simulink model "CCMSysServ"  (R2022b) (continued)
== system CCMSysServ/CCMSysServ_Runnable_sys == (continued)
  SysServCarCfgSts_SysServDUBlowerPwmSignSts_write : Outport  Port=106
      settings: EnsureOutportIsVirtual=on
  SysServCarCfgSts_SysServDUBlowerPwmSts_write : Outport  Port=107
      settings: EnsureOutportIsVirtual=on
  SysServCarCfgSts_SysServDUDCDefrostSts_write : Outport  Port=108
      settings: EnsureOutportIsVirtual=on
  SysServCarCfgSts_SysServDUDCFLMdeSts_write : Outport  Port=109
      settings: EnsureOutportIsVirtual=on
  SysServCarCfgSts_SysServDUDCModeSts_write : Outport  Port=110
      settings: EnsureOutportIsVirtual=on
  SysServCarCfgSts_SysServDUDCRecSts_write : Outport  Port=111
      settings: EnsureOutportIsVirtual=on
  SysServCarCfgSts_SysServDUDCSModeSts_write : Outport  Port=112
      settings: EnsureOutportIsVirtual=on
  SysServCarCfgSts_SysServDUDCSTLSts_write : Outport  Port=113
      settings: EnsureOutportIsVirtual=on
  SysServCarCfgSts_SysServDUDCTLSts_write : Outport  Port=114
      settings: EnsureOutportIsVirtual=on
  SysServCarCfgSts_SysServDUDCTRSts_write : Outport  Port=115
      settings: EnsureOutportIsVirtual=on
  SysServCarCfgSts_SysServDUFLFootSts_write : Outport  Port=116
      settings: EnsureOutportIsVirtual=on
  SysServCarCfgSts_SysServDUFLVentSts_write : Outport  Port=117
      settings: EnsureOutportIsVirtual=on
  SysServCarCfgSts_SysServDUFRFootSts_write : Outport  Port=118
      settings: EnsureOutportIsVirtual=on
  SysServCarCfgSts_SysServDUFRVentSts_write : Outport  Port=119
      settings: EnsureOutportIsVirtual=on
  SysServCarCfgSts_SysServDUIncarMotorSts_write : Outport  Port=120
      settings: EnsureOutportIsVirtual=on
  SysServCarCfgSts_SysServDULin1HBMFSts_write : Outport  Port=121
      settings: EnsureOutportIsVirtual=on
  SysServCarCfgSts_SysServDULin4HFAFSts_write : Outport  Port=122
      settings: EnsureOutportIsVirtual=on
  SysServCarCfgSts_SysServDUSFLFootSts_write : Outport  Port=123
      settings: EnsureOutportIsVirtual=on
  SysServCarCfgSts_SysServDUSFLVentSts_write : Outport  Port=124
      settings: EnsureOutportIsVirtual=on
  SysServCarCfgSts_SysServDUSFRFootSts_write : Outport  Port=125
      settings: EnsureOutportIsVirtual=on
  SysServCarCfgSts_SysServDUSFRVentSts_write : Outport  Port=126
      settings: EnsureOutportIsVirtual=on
  SysServCarCfgSts_SysServDUSnsrForFCmptmtTSts_write : Outport  Port=127
      settings: EnsureOutportIsVirtual=on
  SysServCarCfgSts_SysServDUSnsrForRair_write : Outport  Port=128
      settings: EnsureOutportIsVirtual=on
  SysServCarCfgSts_SysServDUSnsrForRCmptmtTSts_write : Outport  Port=129
      settings: EnsureOutportIsVirtual=on
  SysServEcuSlpDelayFinish_EcuSlpDelayFinishFlg_write : Outport  Port=130
      settings: EnsureOutportIsVirtual=on
  dp_LifeCycle_LifeCycle_write : Outport  Port=131
      settings: EnsureOutportIsVirtual=on
  wires:
  ComMPNCState_PNCState_read/1 -> Terminator_1/1
  ComMPNCState_VFCState_read/1 -> Terminator_2/1
  EcuKeepActv_EcuKeepActv_read/1 -> Terminator_3/1
  CarTiGlb_CarTiGlb_read/1 -> Terminator_4/1
  CarTiGlb_CarTiGlb_IsUpdated_read/1 -> Terminator_5/1
  ActrFrntReqForRowFirstLeModDistbn_ActrFrntReqForRowFirstLeModDistbn_read/1 -> Terminator_6/1
  CarCfg_CarCfg1_read/1 -> Terminator_7/1
  CarCfg_CarCfg10_read/1 -> Terminator_8/1
  CarCfg_CarCfg100_read/1 -> Terminator_9/1
  CarCfg_CarCfg1002_read/1 -> Terminator_10/1
  CarCfg_CarCfg1004_read/1 -> Terminator_11/1
  CarCfg_CarCfg1007_read/1 -> Terminator_12/1
  CarCfg_CarCfg101_read/1 -> Terminator_13/1
  CarCfg_CarCfg1011_read/1 -> Terminator_14/1
  CarCfg_CarCfg1017_read/1 -> Terminator_15/1
  CarCfg_CarCfg1018_read/1 -> Terminator_16/1
  CarCfg_CarCfg102_read/1 -> Terminator_17/1
  CarCfg_CarCfg103_read/1 -> Terminator_18/1
  CarCfg_CarCfg1034_read/1 -> Terminator_19/1
  CarCfg_CarCfg1038_read/1 -> Terminator_20/1
  CarCfg_CarCfg1039_read/1 -> Terminator_21/1
  CarCfg_CarCfg104_read/1 -> Terminator_22/1
  CarCfg_CarCfg1042_read/1 -> Terminator_23/1
  CarCfg_CarCfg1043_read/1 -> Terminator_24/1
  CarCfg_CarCfg1044_read/1 -> Terminator_25/1
  CarCfg_CarCfg1045_read/1 -> Terminator_26/1
  CarCfg_CarCfg1047_read/1 -> Terminator_27/1
  CarCfg_CarCfg105_read/1 -> Terminator_28/1
  CarCfg_CarCfg1052_read/1 -> Terminator_29/1
  CarCfg_CarCfg1057_read/1 -> Terminator_30/1
  CarCfg_CarCfg106_read/1 -> Terminator_31/1
  CarCfg_CarCfg1061_read/1 -> Terminator_32/1
  CarCfg_CarCfg1063_read/1 -> Terminator_33/1
  CarCfg_CarCfg1065_read/1 -> Terminator_34/1
  CarCfg_CarCfg107_read/1 -> Terminator_35/1
  CarCfg_CarCfg1075_read/1 -> Terminator_36/1
  CarCfg_CarCfg1077_read/1 -> Terminator_37/1
  CarCfg_CarCfg108_read/1 -> Terminator_38/1
  CarCfg_CarCfg1080_read/1 -> Terminator_39/1
  CarCfg_CarCfg1083_read/1 -> Terminator_40/1
  CarCfg_CarCfg1087_read/1 -> Terminator_41/1
  CarCfg_CarCfg1088_read/1 -> Terminator_42/1
  CarCfg_CarCfg109_read/1 -> Terminator_43/1
  CarCfg_CarCfg1090_read/1 -> Terminator_44/1
  CarCfg_CarCfg1096_read/1 -> Terminator_45/1
  CarCfg_CarCfg110_read/1 -> Terminator_46/1
  CarCfg_CarCfg1101_read/1 -> Terminator_47/1
  CarCfg_CarCfg1102_read/1 -> Terminator_48/1
  CarCfg_CarCfg1103_read/1 -> Terminator_49/1
  CarCfg_CarCfg1104_read/1 -> Terminator_50/1
  CarCfg_CarCfg111_read/1 -> Terminator_51/1
  CarCfg_CarCfg1111_read/1 -> Terminator_52/1
  CarCfg_CarCfg1112_read/1 -> Terminator_53/1
  CarCfg_CarCfg112_read/1 -> Terminator_54/1
  CarCfg_CarCfg113_read/1 -> Terminator_55/1
  CarCfg_CarCfg1130_read/1 -> Terminator_56/1
  CarCfg_CarCfg1134_read/1 -> Terminator_57/1
  CarCfg_CarCfg1136_read/1 -> Terminator_58/1
  CarCfg_CarCfg114_read/1 -> Terminator_59/1
  CarCfg_CarCfg115_read/1 -> Terminator_60/1
  CarCfg_CarCfg1154_read/1 -> Terminator_61/1
  CarCfg_CarCfg116_read/1 -> Terminator_62/1
  CarCfg_CarCfg1166_read/1 -> Terminator_63/1
  CarCfg_CarCfg117_read/1 -> Terminator_64/1
  CarCfg_CarCfg1170_read/1 -> Terminator_65/1
  CarCfg_CarCfg1171_read/1 -> Terminator_66/1
  CarCfg_CarCfg1172_read/1 -> Terminator_67/1
  CarCfg_CarCfg1173_read/1 -> Terminator_68/1
  CarCfg_CarCfg1174_read/1 -> Terminator_69/1
  CarCfg_CarCfg1175_read/1 -> Terminator_70/1
  CarCfg_CarCfg1177_read/1 -> Terminator_71/1
  CarCfg_CarCfg1178_read/1 -> Terminator_72/1
  CarCfg_CarCfg118_read/1 -> Terminator_73/1
  CarCfg_CarCfg1184_read/1 -> Terminator_74/1
  CarCfg_CarCfg1185_read/1 -> Terminator_75/1
  CarCfg_CarCfg1189_read/1 -> Terminator_76/1
  CarCfg_CarCfg119_read/1 -> Terminator_77/1
  CarCfg_CarCfg12_read/1 -> Terminator_78/1
  CarCfg_CarCfg120_read/1 -> Terminator_79/1
  CarCfg_CarCfg121_read/1 -> Terminator_80/1
  CarCfg_CarCfg1214_read/1 -> Terminator_81/1
  CarCfg_CarCfg1216_read/1 -> Terminator_82/1
  CarCfg_CarCfg1217_read/1 -> Terminator_83/1
  CarCfg_CarCfg1218_read/1 -> Terminator_84/1
  CarCfg_CarCfg1219_read/1 -> Terminator_85/1
  CarCfg_CarCfg122_read/1 -> Terminator_86/1
  CarCfg_CarCfg123_read/1 -> Terminator_87/1
  CarCfg_CarCfg1230_read/1 -> Terminator_88/1
  CarCfg_CarCfg1231_read/1 -> Terminator_89/1
  CarCfg_CarCfg1232_read/1 -> Terminator_90/1
  CarCfg_CarCfg1233_read/1 -> Terminator_91/1
  CarCfg_CarCfg1234_read/1 -> Terminator_92/1
  CarCfg_CarCfg124_read/1 -> Terminator_93/1
  CarCfg_CarCfg1247_read/1 -> Terminator_94/1
  CarCfg_CarCfg125_read/1 -> Terminator_95/1
  CarCfg_CarCfg1250_read/1 -> Terminator_96/1
  CarCfg_CarCfg1251_read/1 -> Terminator_97/1
  CarCfg_CarCfg1258_read/1 -> Terminator_98/1
  CarCfg_CarCfg1259_read/1 -> Terminator_99/1
  CarCfg_CarCfg126_read/1 -> Terminator_100/1
  CarCfg_CarCfg1260_read/1 -> Terminator_101/1
  CarCfg_CarCfg1261_read/1 -> Terminator_102/1
  CarCfg_CarCfg1264_read/1 -> Terminator_103/1
  CarCfg_CarCfg1266_read/1 -> Terminator_104/1
  CarCfg_CarCfg1267_read/1 -> Terminator_105/1
  CarCfg_CarCfg127_read/1 -> Terminator_106/1
  CarCfg_CarCfg1273_read/1 -> Terminator_107/1
  CarCfg_CarCfg1275_read/1 -> Terminator_108/1
  CarCfg_CarCfg1277_read/1 -> Terminator_109/1
  CarCfg_CarCfg1278_read/1 -> Terminator_110/1
  CarCfg_CarCfg1279_read/1 -> Terminator_111/1
  CarCfg_CarCfg128_read/1 -> Terminator_112/1
  CarCfg_CarCfg1283_read/1 -> Terminator_113/1
  CarCfg_CarCfg1285_read/1 -> Terminator_114/1
  CarCfg_CarCfg1286_read/1 -> Terminator_115/1
  CarCfg_CarCfg1287_read/1 -> Terminator_116/1
  CarCfg_CarCfg129_read/1 -> Terminator_117/1
  CarCfg_CarCfg13_read/1 -> Terminator_118/1
  CarCfg_CarCfg130_read/1 -> Terminator_119/1
  CarCfg_CarCfg131_read/1 -> Terminator_120/1
  CarCfg_CarCfg132_read/1 -> Terminator_121/1
  CarCfg_CarCfg133_read/1 -> Terminator_122/1
  CarCfg_CarCfg134_read/1 -> Terminator_123/1
  CarCfg_CarCfg135_read/1 -> Terminator_124/1
  CarCfg_CarCfg136_read/1 -> Terminator_125/1
  CarCfg_CarCfg137_read/1 -> Terminator_126/1
  CarCfg_CarCfg138_read/1 -> Terminator_127/1
  CarCfg_CarCfg139_read/1 -> Terminator_128/1
  CarCfg_CarCfg14_read/1 -> Terminator_129/1
  CarCfg_CarCfg140_read/1 -> Terminator_130/1
  CarCfg_CarCfg141_read/1 -> Terminator_131/1
  CarCfg_CarCfg142_read/1 -> Terminator_132/1
  CarCfg_CarCfg143_read/1 -> Terminator_133/1
  CarCfg_CarCfg144_read/1 -> Terminator_134/1
  CarCfg_CarCfg145_read/1 -> Terminator_135/1
  CarCfg_CarCfg146_read/1 -> Terminator_136/1
  CarCfg_CarCfg148_read/1 -> Terminator_137/1
  CarCfg_CarCfg149_read/1 -> Terminator_138/1
  CarCfg_CarCfg150_read/1 -> Terminator_139/1
  CarCfg_CarCfg151_read/1 -> Terminator_140/1
  CarCfg_CarCfg152_read/1 -> Terminator_141/1
  CarCfg_CarCfg153_read/1 -> Terminator_142/1
  CarCfg_CarCfg154_read/1 -> Terminator_143/1
  CarCfg_CarCfg155_read/1 -> Terminator_144/1
  CarCfg_CarCfg156_read/1 -> Terminator_145/1
  CarCfg_CarCfg157_read/1 -> Terminator_146/1
  CarCfg_CarCfg158_read/1 -> Terminator_147/1
  CarCfg_CarCfg159_read/1 -> Terminator_148/1
  CarCfg_CarCfg16_read/1 -> Terminator_149/1
  CarCfg_CarCfg160_read/1 -> Terminator_150/1
  CarCfg_CarCfg161_read/1 -> Terminator_151/1
  CarCfg_CarCfg162_read/1 -> Terminator_152/1
  CarCfg_CarCfg163_read/1 -> Terminator_153/1
  CarCfg_CarCfg164_read/1 -> Terminator_154/1
  CarCfg_CarCfg165_read/1 -> Terminator_155/1
  CarCfg_CarCfg166_read/1 -> Terminator_156/1
  CarCfg_CarCfg167_read/1 -> Terminator_157/1
  CarCfg_CarCfg168_read/1 -> Terminator_158/1
  CarCfg_CarCfg169_read/1 -> Terminator_159/1
  CarCfg_CarCfg17_read/1 -> Terminator_160/1
  CarCfg_CarCfg170_read/1 -> Terminator_161/1
  CarCfg_CarCfg171_read/1 -> Terminator_162/1
  CarCfg_CarCfg172_read/1 -> Terminator_163/1
  CarCfg_CarCfg173_read/1 -> Terminator_164/1
  CarCfg_CarCfg174_read/1 -> Terminator_165/1
  CarCfg_CarCfg175_read/1 -> Terminator_166/1
  CarCfg_CarCfg176_read/1 -> Terminator_167/1
  CarCfg_CarCfg177_read/1 -> Terminator_168/1
  CarCfg_CarCfg178_read/1 -> Terminator_169/1
  CarCfg_CarCfg179_read/1 -> Terminator_170/1
  CarCfg_CarCfg18_read/1 -> Terminator_171/1
  CarCfg_CarCfg180_read/1 -> Terminator_172/1
  CarCfg_CarCfg181_read/1 -> Terminator_173/1
  CarCfg_CarCfg182_read/1 -> Terminator_174/1
  CarCfg_CarCfg183_read/1 -> Terminator_175/1
  CarCfg_CarCfg184_read/1 -> Terminator_176/1
  CarCfg_CarCfg185_read/1 -> Terminator_177/1
  CarCfg_CarCfg186_read/1 -> Terminator_178/1
  CarCfg_CarCfg187_read/1 -> Terminator_179/1
  CarCfg_CarCfg188_read/1 -> Terminator_180/1
  CarCfg_CarCfg189_read/1 -> Terminator_181/1
  CarCfg_CarCfg19_read/1 -> Terminator_182/1
  CarCfg_CarCfg190_read/1 -> Terminator_183/1
  CarCfg_CarCfg191_read/1 -> Terminator_184/1
  CarCfg_CarCfg192_read/1 -> Terminator_185/1
  CarCfg_CarCfg193_read/1 -> Terminator_186/1
  CarCfg_CarCfg194_read/1 -> Terminator_187/1
  CarCfg_CarCfg195_read/1 -> Terminator_188/1
  CarCfg_CarCfg196_read/1 -> Terminator_189/1
  CarCfg_CarCfg197_read/1 -> Terminator_190/1
  CarCfg_CarCfg198_read/1 -> Terminator_191/1
  CarCfg_CarCfg199_read/1 -> Terminator_192/1
  CarCfg_CarCfg2_read/1 -> Terminator_193/1
  CarCfg_CarCfg20_read/1 -> Terminator_194/1
  CarCfg_CarCfg200_read/1 -> Terminator_195/1
  CarCfg_CarCfg201_read/1 -> Terminator_196/1
  CarCfg_CarCfg202_read/1 -> Terminator_197/1
  CarCfg_CarCfg203_read/1 -> Terminator_198/1
  CarCfg_CarCfg204_read/1 -> Terminator_199/1
  CarCfg_CarCfg205_read/1 -> Terminator_200/1
  CarCfg_CarCfg206_read/1 -> Terminator_201/1
  CarCfg_CarCfg207_read/1 -> Terminator_202/1
  CarCfg_CarCfg208_read/1 -> Terminator_203/1
  CarCfg_CarCfg209_read/1 -> Terminator_204/1
  CarCfg_CarCfg210_read/1 -> Terminator_205/1
  CarCfg_CarCfg211_read/1 -> Terminator_206/1
  CarCfg_CarCfg212_read/1 -> Terminator_207/1
  CarCfg_CarCfg213_read/1 -> Terminator_208/1
  CarCfg_CarCfg214_read/1 -> Terminator_209/1
  CarCfg_CarCfg215_read/1 -> Terminator_210/1
  CarCfg_CarCfg216_read/1 -> Terminator_211/1
  CarCfg_CarCfg217_read/1 -> Terminator_212/1
  CarCfg_CarCfg218_read/1 -> Terminator_213/1
  CarCfg_CarCfg219_read/1 -> Terminator_214/1
  CarCfg_CarCfg22_read/1 -> Terminator_215/1
  CarCfg_CarCfg220_read/1 -> Terminator_216/1
  CarCfg_CarCfg221_read/1 -> Terminator_217/1
  CarCfg_CarCfg222_read/1 -> Terminator_218/1
  CarCfg_CarCfg223_read/1 -> Terminator_219/1
  CarCfg_CarCfg224_read/1 -> Terminator_220/1
  CarCfg_CarCfg225_read/1 -> Terminator_221/1
  CarCfg_CarCfg226_read/1 -> Terminator_222/1
  CarCfg_CarCfg227_read/1 -> Terminator_223/1
  CarCfg_CarCfg228_read/1 -> Terminator_224/1
  CarCfg_CarCfg229_read/1 -> Terminator_225/1
  CarCfg_CarCfg23_read/1 -> Terminator_226/1
  CarCfg_CarCfg230_read/1 -> Terminator_227/1
  CarCfg_CarCfg231_read/1 -> Terminator_228/1
  CarCfg_CarCfg232_read/1 -> Terminator_229/1
  CarCfg_CarCfg235_read/1 -> Terminator_230/1
  CarCfg_CarCfg236_read/1 -> Terminator_231/1
  CarCfg_CarCfg237_read/1 -> Terminator_232/1
  CarCfg_CarCfg238_read/1 -> Terminator_233/1
  CarCfg_CarCfg239_read/1 -> Terminator_234/1
  CarCfg_CarCfg24_read/1 -> Terminator_235/1
  CarCfg_CarCfg240_read/1 -> Terminator_236/1
  CarCfg_CarCfg241_read/1 -> Terminator_237/1
  CarCfg_CarCfg242_read/1 -> Terminator_238/1
  CarCfg_CarCfg243_read/1 -> Terminator_239/1
  CarCfg_CarCfg244_read/1 -> Terminator_240/1
  CarCfg_CarCfg245_read/1 -> Terminator_241/1
  CarCfg_CarCfg246_read/1 -> Terminator_242/1
  CarCfg_CarCfg247_read/1 -> Terminator_243/1
  CarCfg_CarCfg248_read/1 -> Terminator_244/1
  CarCfg_CarCfg249_read/1 -> Terminator_245/1
  CarCfg_CarCfg25_read/1 -> Terminator_246/1
  CarCfg_CarCfg250_read/1 -> Terminator_247/1
  CarCfg_CarCfg253_read/1 -> Terminator_248/1
  CarCfg_CarCfg254_read/1 -> Terminator_249/1
  CarCfg_CarCfg255_read/1 -> Terminator_250/1
  CarCfg_CarCfg256_read/1 -> Terminator_251/1
  CarCfg_CarCfg257_read/1 -> Terminator_252/1
  CarCfg_CarCfg258_read/1 -> Terminator_253/1
  CarCfg_CarCfg259_read/1 -> Terminator_254/1
  CarCfg_CarCfg262_read/1 -> Terminator_255/1
  CarCfg_CarCfg263_read/1 -> Terminator_256/1
  CarCfg_CarCfg267_read/1 -> Terminator_257/1
  CarCfg_CarCfg268_read/1 -> Terminator_258/1
  CarCfg_CarCfg270_read/1 -> Terminator_259/1
  CarCfg_CarCfg271_read/1 -> Terminator_260/1
  CarCfg_CarCfg273_read/1 -> Terminator_261/1
  CarCfg_CarCfg274_read/1 -> Terminator_262/1
  CarCfg_CarCfg275_read/1 -> Terminator_263/1
  CarCfg_CarCfg28_read/1 -> Terminator_264/1
  CarCfg_CarCfg289_read/1 -> Terminator_265/1
  CarCfg_CarCfg290_read/1 -> Terminator_266/1
  CarCfg_CarCfg3_read/1 -> Terminator_267/1
  CarCfg_CarCfg30_read/1 -> Terminator_268/1
  CarCfg_CarCfg301_read/1 -> Terminator_269/1
  CarCfg_CarCfg302_read/1 -> Terminator_270/1
  CarCfg_CarCfg303_read/1 -> Terminator_271/1
  CarCfg_CarCfg304_read/1 -> Terminator_272/1
  CarCfg_CarCfg305_read/1 -> Terminator_273/1
  CarCfg_CarCfg307_read/1 -> Terminator_274/1
  CarCfg_CarCfg308_read/1 -> Terminator_275/1
  CarCfg_CarCfg309_read/1 -> Terminator_276/1
  CarCfg_CarCfg310_read/1 -> Terminator_277/1
  CarCfg_CarCfg311_read/1 -> Terminator_278/1
  CarCfg_CarCfg312_read/1 -> Terminator_279/1
  CarCfg_CarCfg313_read/1 -> Terminator_280/1
  CarCfg_CarCfg314_read/1 -> Terminator_281/1
  CarCfg_CarCfg315_read/1 -> Terminator_282/1
  CarCfg_CarCfg316_read/1 -> Terminator_283/1
  CarCfg_CarCfg317_read/1 -> Terminator_284/1
  CarCfg_CarCfg318_read/1 -> Terminator_285/1
  CarCfg_CarCfg319_read/1 -> Terminator_286/1
  CarCfg_CarCfg32_read/1 -> Terminator_287/1
  CarCfg_CarCfg321_read/1 -> Terminator_288/1
  CarCfg_CarCfg322_read/1 -> Terminator_289/1
  CarCfg_CarCfg323_read/1 -> Terminator_290/1
  CarCfg_CarCfg324_read/1 -> Terminator_291/1
  CarCfg_CarCfg325_read/1 -> Terminator_292/1
  CarCfg_CarCfg33_read/1 -> Terminator_293/1
  CarCfg_CarCfg335_read/1 -> Terminator_294/1
  CarCfg_CarCfg336_read/1 -> Terminator_295/1
  CarCfg_CarCfg337_read/1 -> Terminator_296/1
  CarCfg_CarCfg338_read/1 -> Terminator_297/1
  CarCfg_CarCfg339_read/1 -> Terminator_298/1
  CarCfg_CarCfg34_read/1 -> Terminator_299/1
  CarCfg_CarCfg340_read/1 -> Terminator_300/1
  CarCfg_CarCfg341_read/1 -> Terminator_301/1
  CarCfg_CarCfg342_read/1 -> Terminator_302/1
  CarCfg_CarCfg343_read/1 -> Terminator_303/1
  CarCfg_CarCfg344_read/1 -> Terminator_304/1
  CarCfg_CarCfg345_read/1 -> Terminator_305/1
  CarCfg_CarCfg346_read/1 -> Terminator_306/1
  CarCfg_CarCfg347_read/1 -> Terminator_307/1
  CarCfg_CarCfg348_read/1 -> Terminator_308/1
  CarCfg_CarCfg349_read/1 -> Terminator_309/1
  CarCfg_CarCfg35_read/1 -> Terminator_310/1
  CarCfg_CarCfg350_read/1 -> Terminator_311/1
  CarCfg_CarCfg351_read/1 -> Terminator_312/1
  CarCfg_CarCfg352_read/1 -> Terminator_313/1
  CarCfg_CarCfg353_read/1 -> Terminator_314/1
  CarCfg_CarCfg354_read/1 -> Terminator_315/1
  CarCfg_CarCfg355_read/1 -> Terminator_316/1
  CarCfg_CarCfg356_read/1 -> Terminator_317/1
  CarCfg_CarCfg357_read/1 -> Terminator_318/1
  CarCfg_CarCfg358_read/1 -> Terminator_319/1
  CarCfg_CarCfg359_read/1 -> Terminator_320/1
  CarCfg_CarCfg36_read/1 -> Terminator_321/1
  CarCfg_CarCfg360_read/1 -> Terminator_322/1
  CarCfg_CarCfg361_read/1 -> Terminator_323/1
  CarCfg_CarCfg362_read/1 -> Terminator_324/1
  CarCfg_CarCfg363_read/1 -> Terminator_325/1
  CarCfg_CarCfg364_read/1 -> Terminator_326/1
  CarCfg_CarCfg365_read/1 -> Terminator_327/1
  CarCfg_CarCfg366_read/1 -> Terminator_328/1
  CarCfg_CarCfg367_read/1 -> Terminator_329/1
  CarCfg_CarCfg368_read/1 -> Terminator_330/1
  CarCfg_CarCfg369_read/1 -> Terminator_331/1
  CarCfg_CarCfg37_read/1 -> Terminator_332/1
  CarCfg_CarCfg371_read/1 -> Terminator_333/1
  CarCfg_CarCfg372_read/1 -> Terminator_334/1
  CarCfg_CarCfg373_read/1 -> Terminator_335/1
  CarCfg_CarCfg374_read/1 -> Terminator_336/1
  CarCfg_CarCfg376_read/1 -> Terminator_337/1
  CarCfg_CarCfg38_read/1 -> Terminator_338/1
  CarCfg_CarCfg39_read/1 -> Terminator_339/1
  CarCfg_CarCfg4_read/1 -> Terminator_340/1
  CarCfg_CarCfg401_read/1 -> Terminator_341/1
  CarCfg_CarCfg407_read/1 -> Terminator_342/1
  CarCfg_CarCfg408_read/1 -> Terminator_343/1
  CarCfg_CarCfg409_read/1 -> Terminator_344/1
  CarCfg_CarCfg41_read/1 -> Terminator_345/1
  CarCfg_CarCfg410_read/1 -> Terminator_346/1
  CarCfg_CarCfg413_read/1 -> Terminator_347/1
  CarCfg_CarCfg414_read/1 -> Terminator_348/1
  CarCfg_CarCfg415_read/1 -> Terminator_349/1
  CarCfg_CarCfg42_read/1 -> Terminator_350/1
  CarCfg_CarCfg43_read/1 -> Terminator_351/1
  CarCfg_CarCfg44_read/1 -> Terminator_352/1
  CarCfg_CarCfg441_read/1 -> Terminator_353/1
  CarCfg_CarCfg449_read/1 -> Terminator_354/1
  CarCfg_CarCfg45_read/1 -> Terminator_355/1
  CarCfg_CarCfg450_read/1 -> Terminator_356/1
  CarCfg_CarCfg451_read/1 -> Terminator_357/1
  CarCfg_CarCfg452_read/1 -> Terminator_358/1
  CarCfg_CarCfg453_read/1 -> Terminator_359/1
  CarCfg_CarCfg454_read/1 -> Terminator_360/1
  CarCfg_CarCfg455_read/1 -> Terminator_361/1
  CarCfg_CarCfg456_read/1 -> Terminator_362/1
  CarCfg_CarCfg457_read/1 -> Terminator_363/1
  CarCfg_CarCfg458_read/1 -> Terminator_364/1
  CarCfg_CarCfg459_read/1 -> Terminator_365/1
  CarCfg_CarCfg46_read/1 -> Terminator_366/1
  CarCfg_CarCfg460_read/1 -> Terminator_367/1
  CarCfg_CarCfg461_read/1 -> Terminator_368/1
  CarCfg_CarCfg462_read/1 -> Terminator_369/1
  CarCfg_CarCfg463_read/1 -> Terminator_370/1
  CarCfg_CarCfg464_read/1 -> Terminator_371/1
  CarCfg_CarCfg465_read/1 -> Terminator_372/1
  CarCfg_CarCfg466_read/1 -> Terminator_373/1
  CarCfg_CarCfg467_read/1 -> Terminator_374/1
  CarCfg_CarCfg468_read/1 -> Terminator_375/1
  CarCfg_CarCfg469_read/1 -> Terminator_376/1
  CarCfg_CarCfg47_read/1 -> Terminator_377/1
  CarCfg_CarCfg470_read/1 -> Terminator_378/1
  CarCfg_CarCfg471_read/1 -> Terminator_379/1
  CarCfg_CarCfg472_read/1 -> Terminator_380/1
  CarCfg_CarCfg473_read/1 -> Terminator_381/1
  CarCfg_CarCfg474_read/1 -> Terminator_382/1
  CarCfg_CarCfg475_read/1 -> Terminator_383/1
  CarCfg_CarCfg476_read/1 -> Terminator_384/1
  CarCfg_CarCfg477_read/1 -> Terminator_385/1
  CarCfg_CarCfg478_read/1 -> Terminator_386/1
  CarCfg_CarCfg479_read/1 -> Terminator_387/1
  CarCfg_CarCfg48_read/1 -> Terminator_388/1
  CarCfg_CarCfg480_read/1 -> Terminator_389/1
  CarCfg_CarCfg481_read/1 -> Terminator_390/1
  CarCfg_CarCfg482_read/1 -> Terminator_391/1
  CarCfg_CarCfg483_read/1 -> Terminator_392/1
  CarCfg_CarCfg484_read/1 -> Terminator_393/1
  CarCfg_CarCfg485_read/1 -> Terminator_394/1
  CarCfg_CarCfg486_read/1 -> Terminator_395/1
  CarCfg_CarCfg487_read/1 -> Terminator_396/1
  CarCfg_CarCfg488_read/1 -> Terminator_397/1
  CarCfg_CarCfg489_read/1 -> Terminator_398/1
  CarCfg_CarCfg49_read/1 -> Terminator_399/1
  CarCfg_CarCfg490_read/1 -> Terminator_400/1
  CarCfg_CarCfg491_read/1 -> Terminator_401/1
  CarCfg_CarCfg493_read/1 -> Terminator_402/1
  CarCfg_CarCfg494_read/1 -> Terminator_403/1
  CarCfg_CarCfg495_read/1 -> Terminator_404/1
  CarCfg_CarCfg496_read/1 -> Terminator_405/1
  CarCfg_CarCfg497_read/1 -> Terminator_406/1
  CarCfg_CarCfg498_read/1 -> Terminator_407/1
  CarCfg_CarCfg499_read/1 -> Terminator_408/1
  CarCfg_CarCfg5_read/1 -> Terminator_409/1
  CarCfg_CarCfg50_read/1 -> Terminator_410/1
  CarCfg_CarCfg500_read/1 -> Terminator_411/1
  CarCfg_CarCfg501_read/1 -> Terminator_412/1
  CarCfg_CarCfg502_read/1 -> Terminator_413/1
  CarCfg_CarCfg503_read/1 -> Terminator_414/1
  CarCfg_CarCfg504_read/1 -> Terminator_415/1
  CarCfg_CarCfg505_read/1 -> Terminator_416/1
  CarCfg_CarCfg506_read/1 -> Terminator_417/1
  CarCfg_CarCfg507_read/1 -> Terminator_418/1
  CarCfg_CarCfg508_read/1 -> Terminator_419/1
  CarCfg_CarCfg509_read/1 -> Terminator_420/1
  CarCfg_CarCfg51_read/1 -> Terminator_421/1
  CarCfg_CarCfg510_read/1 -> Terminator_422/1
  CarCfg_CarCfg511_read/1 -> Terminator_423/1
  CarCfg_CarCfg512_read/1 -> Terminator_424/1
  CarCfg_CarCfg513_read/1 -> Terminator_425/1
  CarCfg_CarCfg514_read/1 -> Terminator_426/1
  CarCfg_CarCfg515_read/1 -> Terminator_427/1
  CarCfg_CarCfg516_read/1 -> Terminator_428/1
  CarCfg_CarCfg517_read/1 -> Terminator_429/1
  CarCfg_CarCfg518_read/1 -> Terminator_430/1
  CarCfg_CarCfg519_read/1 -> Terminator_431/1
  CarCfg_CarCfg52_read/1 -> Terminator_432/1
  CarCfg_CarCfg520_read/1 -> Terminator_433/1
  CarCfg_CarCfg521_read/1 -> Terminator_434/1
  CarCfg_CarCfg522_read/1 -> Terminator_435/1
  CarCfg_CarCfg523_read/1 -> Terminator_436/1
  CarCfg_CarCfg524_read/1 -> Terminator_437/1
  CarCfg_CarCfg525_read/1 -> Terminator_438/1
  CarCfg_CarCfg526_read/1 -> Terminator_439/1
  CarCfg_CarCfg527_read/1 -> Terminator_440/1
  CarCfg_CarCfg528_read/1 -> Terminator_441/1
  CarCfg_CarCfg529_read/1 -> Terminator_442/1
  CarCfg_CarCfg53_read/1 -> Terminator_443/1
  CarCfg_CarCfg531_read/1 -> Terminator_444/1
  CarCfg_CarCfg532_read/1 -> Terminator_445/1
  CarCfg_CarCfg533_read/1 -> Terminator_446/1
  CarCfg_CarCfg534_read/1 -> Terminator_447/1
  CarCfg_CarCfg535_read/1 -> Terminator_448/1
  CarCfg_CarCfg536_read/1 -> Terminator_449/1
  CarCfg_CarCfg54_read/1 -> Terminator_450/1
  CarCfg_CarCfg540_read/1 -> Terminator_451/1
  CarCfg_CarCfg541_read/1 -> Terminator_452/1
  CarCfg_CarCfg543_read/1 -> Terminator_453/1
  CarCfg_CarCfg544_read/1 -> Terminator_454/1
  CarCfg_CarCfg545_read/1 -> Terminator_455/1
  CarCfg_CarCfg546_read/1 -> Terminator_456/1
  CarCfg_CarCfg547_read/1 -> Terminator_457/1
  CarCfg_CarCfg55_read/1 -> Terminator_458/1
  CarCfg_CarCfg550_read/1 -> Terminator_459/1
  CarCfg_CarCfg551_read/1 -> Terminator_460/1
  CarCfg_CarCfg56_read/1 -> Terminator_461/1
  CarCfg_CarCfg561_read/1 -> Terminator_462/1
  CarCfg_CarCfg564_read/1 -> Terminator_463/1
  CarCfg_CarCfg565_read/1 -> Terminator_464/1
  CarCfg_CarCfg566_read/1 -> Terminator_465/1
  CarCfg_CarCfg567_read/1 -> Terminator_466/1
  CarCfg_CarCfg568_read/1 -> Terminator_467/1
  CarCfg_CarCfg569_read/1 -> Terminator_468/1
  CarCfg_CarCfg57_read/1 -> Terminator_469/1
  CarCfg_CarCfg578_read/1 -> Terminator_470/1
  CarCfg_CarCfg579_read/1 -> Terminator_471/1
  CarCfg_CarCfg58_read/1 -> Terminator_472/1
  CarCfg_CarCfg580_read/1 -> Terminator_473/1
  CarCfg_CarCfg59_read/1 -> Terminator_474/1
  CarCfg_CarCfg590_read/1 -> Terminator_475/1
  CarCfg_CarCfg591_read/1 -> Terminator_476/1
  CarCfg_CarCfg593_read/1 -> Terminator_477/1
  CarCfg_CarCfg595_read/1 -> Terminator_478/1
  CarCfg_CarCfg599_read/1 -> Terminator_479/1
  CarCfg_CarCfg6_read/1 -> Terminator_480/1
  CarCfg_CarCfg60_read/1 -> Terminator_481/1
  CarCfg_CarCfg604_read/1 -> Terminator_482/1
  CarCfg_CarCfg609_read/1 -> Terminator_483/1
  CarCfg_CarCfg61_read/1 -> Terminator_484/1
  CarCfg_CarCfg611_read/1 -> Terminator_485/1
  CarCfg_CarCfg613_read/1 -> Terminator_486/1
  CarCfg_CarCfg615_read/1 -> Terminator_487/1
  CarCfg_CarCfg616_read/1 -> Terminator_488/1
  CarCfg_CarCfg617_read/1 -> Terminator_489/1
  CarCfg_CarCfg618_read/1 -> Terminator_490/1
  CarCfg_CarCfg62_read/1 -> Terminator_491/1
  CarCfg_CarCfg622_read/1 -> Terminator_492/1
  CarCfg_CarCfg624_read/1 -> Terminator_493/1
  CarCfg_CarCfg626_read/1 -> Terminator_494/1
  CarCfg_CarCfg627_read/1 -> Terminator_495/1
  CarCfg_CarCfg628_read/1 -> Terminator_496/1
  CarCfg_CarCfg629_read/1 -> Terminator_497/1
  CarCfg_CarCfg63_read/1 -> Terminator_498/1
  CarCfg_CarCfg630_read/1 -> Terminator_499/1
  CarCfg_CarCfg634_read/1 -> Terminator_500/1
  CarCfg_CarCfg635_read/1 -> Terminator_501/1
  CarCfg_CarCfg639_read/1 -> Terminator_502/1
  CarCfg_CarCfg64_read/1 -> Terminator_503/1
  CarCfg_CarCfg640_read/1 -> Terminator_504/1
  CarCfg_CarCfg642_read/1 -> Terminator_505/1
  CarCfg_CarCfg644_read/1 -> Terminator_506/1
  CarCfg_CarCfg645_read/1 -> Terminator_507/1
  CarCfg_CarCfg646_read/1 -> Terminator_508/1
  CarCfg_CarCfg647_read/1 -> Terminator_509/1
  CarCfg_CarCfg648_read/1 -> Terminator_510/1
  CarCfg_CarCfg65_read/1 -> Terminator_511/1
  CarCfg_CarCfg650_read/1 -> Terminator_512/1
  CarCfg_CarCfg651_read/1 -> Terminator_513/1
  CarCfg_CarCfg652_read/1 -> Terminator_514/1
  CarCfg_CarCfg659_read/1 -> Terminator_515/1
  CarCfg_CarCfg66_read/1 -> Terminator_516/1
  CarCfg_CarCfg660_read/1 -> Terminator_517/1
  CarCfg_CarCfg665_read/1 -> Terminator_518/1
  CarCfg_CarCfg67_read/1 -> Terminator_519/1
  CarCfg_CarCfg671_read/1 -> Terminator_520/1
  CarCfg_CarCfg672_read/1 -> Terminator_521/1
  CarCfg_CarCfg675_read/1 -> Terminator_522/1
  CarCfg_CarCfg677_read/1 -> Terminator_523/1
  CarCfg_CarCfg678_read/1 -> Terminator_524/1
  CarCfg_CarCfg68_read/1 -> Terminator_525/1
  CarCfg_CarCfg683_read/1 -> Terminator_526/1
  CarCfg_CarCfg684_read/1 -> Terminator_527/1
  CarCfg_CarCfg686_read/1 -> Terminator_528/1
  CarCfg_CarCfg687_read/1 -> Terminator_529/1
  CarCfg_CarCfg688_read/1 -> Terminator_530/1
  CarCfg_CarCfg69_read/1 -> Terminator_531/1
  CarCfg_CarCfg695_read/1 -> Terminator_532/1
  CarCfg_CarCfg697_read/1 -> Terminator_533/1
  CarCfg_CarCfg698_read/1 -> Terminator_534/1
  CarCfg_CarCfg699_read/1 -> Terminator_535/1
  CarCfg_CarCfg70_read/1 -> Terminator_536/1
  CarCfg_CarCfg701_read/1 -> Terminator_537/1
  CarCfg_CarCfg705_read/1 -> Terminator_538/1
  CarCfg_CarCfg709_read/1 -> Terminator_539/1
  CarCfg_CarCfg71_read/1 -> Terminator_540/1
  CarCfg_CarCfg714_read/1 -> Terminator_541/1
  CarCfg_CarCfg715_read/1 -> Terminator_542/1
  CarCfg_CarCfg718_read/1 -> Terminator_543/1
  CarCfg_CarCfg719_read/1 -> Terminator_544/1
  CarCfg_CarCfg72_read/1 -> Terminator_545/1
  CarCfg_CarCfg720_read/1 -> Terminator_546/1
  CarCfg_CarCfg721_read/1 -> Terminator_547/1
  CarCfg_CarCfg726_read/1 -> Terminator_548/1
  CarCfg_CarCfg73_read/1 -> Terminator_549/1
  CarCfg_CarCfg732_read/1 -> Terminator_550/1
  CarCfg_CarCfg733_read/1 -> Terminator_551/1
  CarCfg_CarCfg734_read/1 -> Terminator_552/1
  CarCfg_CarCfg74_read/1 -> Terminator_553/1
  CarCfg_CarCfg740_read/1 -> Terminator_554/1
  CarCfg_CarCfg741_read/1 -> Terminator_555/1
  CarCfg_CarCfg742_read/1 -> Terminator_556/1
  CarCfg_CarCfg746_read/1 -> Terminator_557/1
  CarCfg_CarCfg75_read/1 -> Terminator_558/1
  CarCfg_CarCfg753_read/1 -> Terminator_559/1
  CarCfg_CarCfg755_read/1 -> Terminator_560/1
  CarCfg_CarCfg756_read/1 -> Terminator_561/1
  CarCfg_CarCfg76_read/1 -> Terminator_562/1
  CarCfg_CarCfg763_read/1 -> Terminator_563/1
  CarCfg_CarCfg766_read/1 -> Terminator_564/1
  CarCfg_CarCfg768_read/1 -> Terminator_565/1
  CarCfg_CarCfg77_read/1 -> Terminator_566/1
  CarCfg_CarCfg770_read/1 -> Terminator_567/1
  CarCfg_CarCfg773_read/1 -> Terminator_568/1
  CarCfg_CarCfg776_read/1 -> Terminator_569/1
  CarCfg_CarCfg779_read/1 -> Terminator_570/1
  CarCfg_CarCfg78_read/1 -> Terminator_571/1
  CarCfg_CarCfg787_read/1 -> Terminator_572/1
  CarCfg_CarCfg789_read/1 -> Terminator_573/1
  CarCfg_CarCfg79_read/1 -> Terminator_574/1
  CarCfg_CarCfg790_read/1 -> Terminator_575/1
  CarCfg_CarCfg793_read/1 -> Terminator_576/1
  CarCfg_CarCfg794_read/1 -> Terminator_577/1
  CarCfg_CarCfg798_read/1 -> Terminator_578/1
  CarCfg_CarCfg8_read/1 -> Terminator_579/1
  CarCfg_CarCfg80_read/1 -> Terminator_580/1
  CarCfg_CarCfg81_read/1 -> Terminator_581/1
  CarCfg_CarCfg811_read/1 -> Terminator_582/1
  CarCfg_CarCfg814_read/1 -> Terminator_583/1
  CarCfg_CarCfg816_read/1 -> Terminator_584/1
  CarCfg_CarCfg82_read/1 -> Terminator_585/1
  CarCfg_CarCfg822_read/1 -> Terminator_586/1
  CarCfg_CarCfg826_read/1 -> Terminator_587/1
  CarCfg_CarCfg829_read/1 -> Terminator_588/1
  CarCfg_CarCfg83_read/1 -> Terminator_589/1
  CarCfg_CarCfg831_read/1 -> Terminator_590/1
  CarCfg_CarCfg834_read/1 -> Terminator_591/1
  CarCfg_CarCfg835_read/1 -> Terminator_592/1
  CarCfg_CarCfg837_read/1 -> Terminator_593/1
  CarCfg_CarCfg839_read/1 -> Terminator_594/1
  CarCfg_CarCfg84_read/1 -> Terminator_595/1
  CarCfg_CarCfg841_read/1 -> Terminator_596/1
  CarCfg_CarCfg842_read/1 -> Terminator_597/1
  CarCfg_CarCfg846_read/1 -> Terminator_598/1
  CarCfg_CarCfg848_read/1 -> Terminator_599/1
  CarCfg_CarCfg849_read/1 -> Terminator_600/1
  CarCfg_CarCfg85_read/1 -> Terminator_601/1
  CarCfg_CarCfg851_read/1 -> Terminator_602/1
  CarCfg_CarCfg855_read/1 -> Terminator_603/1
  CarCfg_CarCfg857_read/1 -> Terminator_604/1
  CarCfg_CarCfg858_read/1 -> Terminator_605/1
  CarCfg_CarCfg86_read/1 -> Terminator_606/1
  CarCfg_CarCfg87_read/1 -> Terminator_607/1
  CarCfg_CarCfg88_read/1 -> Terminator_608/1
  CarCfg_CarCfg89_read/1 -> Terminator_609/1
  CarCfg_CarCfg9_read/1 -> Terminator_610/1
  CarCfg_CarCfg90_read/1 -> Terminator_611/1
  CarCfg_CarCfg91_read/1 -> Terminator_612/1
  CarCfg_CarCfg92_read/1 -> Terminator_613/1
  CarCfg_CarCfg93_read/1 -> Terminator_614/1
  CarCfg_CarCfg94_read/1 -> Terminator_615/1
  CarCfg_CarCfg95_read/1 -> Terminator_616/1
  CarCfg_CarCfg96_read/1 -> Terminator_617/1
  CarCfg_CarCfg97_read/1 -> Terminator_618/1
  CarCfg_CarCfg98_read/1 -> Terminator_619/1
  CarCfg_CarCfg99_read/1 -> Terminator_620/1
  VehCfgPrm_VehCfgPrm_read/1 -> Terminator_621/1
  VehCfgPrmExt_VehCfgPrmExt_read/1 -> Terminator_622/1
  VehModMngtGlbSafe1_VehModMngtGlbSafe1_read/1 -> Terminator_623/1
  Vin_Vin_read/1 -> Terminator_624/1
  BswLifeCycle_LifeCycle_read/1 -> Terminator_625/1
  PA_NvMBlockCarCfgDataInit/1 -> Terminator_626/1
  PA_NvMBlockDidDataD005Init/1 -> Terminator_627/1
  PA_NvMBlockDidDataD09AInit/1 -> Terminator_628/1
  PA_NvMBlockDidDataE103Init/1 -> Terminator_629/1
  PA_NvMBlockDidDataF190Init/1 -> Terminator_630/1
  PA_NvMBlockDidDataF1ABInit/1 -> Terminator_631/1
  Ground_1/1 -> CarCfgForClimaCtrl_CarCfgFor3RDROWSEATHEAT_write/1
  Ground_2/1 -> CarCfgForClimaCtrl_CarCfgFor3RDROWSEATVENTILATED_write/1
  Ground_3/1 -> CarCfgForClimaCtrl_CarCfgForBRAND_write/1
  Ground_4/1 -> CarCfgForClimaCtrl_CarCfgForCabinOxgenSupply_write/1
  Ground_5/1 -> CarCfgForClimaCtrl_CarCfgForElectricLiftingModule_write/1
  Ground_6/1 -> CarCfgForClimaCtrl_CarCfgForExtVentnClimaCtrl_write/1
  Ground_7/1 -> CarCfgForClimaCtrl_CarCfgForTEMPEREDSTORAGEBOX_write/1
  Ground_8/1 -> CarCfgForClimaCtrl_CarCfgForFloatingDriving_write/1
  Ground_9/1 -> CarCfgForClimaCtrl_CarCfgForInteriorViewCamera_write/1
  Ground_10/1 -> CarCfgForClimaCtrl_CarCfgForLifeDetection_write/1
  Ground_11/1 -> CarCfgForClimaCtrl_CarCfgForMARKET_write/1
  Ground_12/1 -> CarCfgForClimaCtrl_CarCfgForPowerSeats_write/1
  Ground_13/1 -> CarCfgForClimaCtrl_CarCfgForPTCInFrontHVAC_write/1
  Ground_14/1 -> CarCfgForClimaCtrl_CarCfgForRFKEY_write/1
  Ground_15/1 -> CarCfgForClimaCtrl_CarCfgForVentLiftMotorExiSts_write/1
  Ground_16/1 -> CarCfgForClimaCtrl_CarCfgForWadingAssistSystem_write/1
  Ground_17/1 -> CarCfgForClimaCtrl_CarCfgWindFollowHumanMov_write/1
  Ground_18/1 -> HvacCfgForClimaCtrl_HvacCfgForAQSTypes_write/1
  Ground_19/1 -> HvacCfgForClimaCtrl_HvacCfgForHvacLowSideRETXVESts_write/1
  Ground_20/1 -> HvacCfgForClimaCtrl_HvacCfgForHvacReBlowerType_write/1
  Ground_21/1 -> HvacCfgForClimaCtrl_HvacCfgForHvacReVentPstn_write/1
  Ground_22/1 -> HvacCfgForClimaCtrl_HvacCfgForHvacSnsrRCmptmtESts_write/1
  Ground_23/1 -> HvacCfgForClimaCtrl_HvacCfgForHvacSnsrReHexESts_write/1
  Ground_24/1 -> HvacCfgReqVersionReplace_HvacCfgReqVersionReplace_write/1
  Ground_25/1 -> SysServCarCfgSts_SysServCCPLin2AQSSts_write/1
  Ground_26/1 -> SysServCarCfgSts_SysServDUBlowerPwmSignTypeSts_write/1
  Ground_27/1 -> SysServCarCfgSts_SysServDULin4HFAFStsH_write/1
  Ground_28/1 -> SysServCarCfgSts_SysServDUReBlowerPwmSignSts_write/1
  Ground_29/1 -> SysServCarCfgSts_SysServDUReBlowerPwmSts_write/1
  Ground_30/1 -> SysServCarCfgSts_SysServFloatingMotorSts_write/1
  Ground_31/1 -> SysServCarCfgSts_SysServFrntHEXSnsrPosn_write/1
  Ground_32/1 -> SysServCarCfgSts_SysServLowSideRETXVSts_write/1
  Ground_33/1 -> SysServCarCfgSts_SysServReHEXSnsrPosn_write/1
  Ground_34/1 -> SysServCarCfgSts_SysServRHFASts_write/1
  Ground_35/1 -> SysServCarCfgSts_SysServSnsrForFCmptmtTType_write/1
  Ground_36/1 -> CarCfgForClimaCtrl_CarCfgForAutDemst_write/1
  Ground_37/1 -> CarCfgForClimaCtrl_CarCfgForAirClng_write/1
  Ground_38/1 -> CarCfgForClimaCtrl_CarCfgForAirClnrAdv_write/1
  Ground_39/1 -> CarCfgForClimaCtrl_CarCfgForAirFrag_write/1
  Ground_40/1 -> CarCfgForClimaCtrl_CarCfgForAirMtrlSnsr_write/1
  Ground_41/1 -> CarCfgForClimaCtrl_CarCfgForAirQly_write/1
  Ground_42/1 -> CarCfgForClimaCtrl_CarCfgForAmntOfSeat_write/1
  Ground_43/1 -> CarCfgForClimaCtrl_CarCfgForAuxWtrPmp_write/1
  Ground_44/1 -> CarCfgForClimaCtrl_CarCfgForBypVlvInHvac_write/1
  Ground_45/1 -> CarCfgForClimaCtrl_CarCfgForClsVlvInHvac_write/1
  Ground_46/1 -> CarCfgForClimaCtrl_CarCfgForCmprAcTyp_write/1
  Ground_47/1 -> CarCfgForClimaCtrl_CarCfgForCmptmtClng_write/1
  Ground_48/1 -> CarCfgForClimaCtrl_CarCfgForElecVentAdjmt_write/1
  Ground_49/1 -> CarCfgForClimaCtrl_CarCfgForFlcTyp_write/1
  Ground_50/1 -> CarCfgForClimaCtrl_CarCfgForFoldCSD_write/1
  Ground_51/1 -> CarCfgForClimaCtrl_CarCfgForFuTyp_write/1
  Ground_52/1 -> CarCfgForClimaCtrl_CarCfgForGlassTyp_write/1
  Ground_53/1 -> CarCfgForClimaCtrl_CarCfgForHeadRadar_write/1
  Ground_54/1 -> CarCfgForClimaCtrl_CarCfgForHeatPmp_write/1
  Ground_55/1 -> CarCfgForClimaCtrl_CarCfgForHeatrDurgDrvg_write/1
  Ground_56/1 -> CarCfgForClimaCtrl_CarCfgForHeatrTyp_write/1
  Ground_57/1 -> CarCfgForClimaCtrl_CarCfgForHvacFilTyp_write/1
  Ground_58/1 -> CarCfgForClimaCtrl_CarCfgForHvacFlrSnsrForT_write/1
  Ground_59/1 -> CarCfgForClimaCtrl_CarCfgForHvacHeatrSnsrT_write/1
  Ground_60/1 -> CarCfgForClimaCtrl_CarCfgForHvacRe_write/1
  Ground_61/1 -> CarCfgForClimaCtrl_CarCfgForHvacTyp_write/1
  Ground_62/1 -> CarCfgForClimaCtrl_CarCfgForLoVoltElecCfg_write/1
  Ground_63/1 -> CarCfgForClimaCtrl_CarCfgForNavSysandIntnmap_write/1
  Ground_64/1 -> CarCfgForClimaCtrl_CarCfgForNrOfDoor_write/1
  Ground_65/1 -> CarCfgForClimaCtrl_CarCfgForPipeInFuTank_write/1
  Ground_66/1 -> CarCfgForClimaCtrl_CarCfgForPreClng_write/1
  Ground_67/1 -> CarCfgForClimaCtrl_CarCfgForPrkgCDSNotAvl_write/1
  Ground_68/1 -> CarCfgForClimaCtrl_CarCfgForPrkgClima_write/1
  Ground_69/1 -> CarCfgForClimaCtrl_CarCfgForPrpsnTyp_write/1
  Ground_70/1 -> CarCfgForClimaCtrl_CarCfgForRemEngStrtPreCdn_write/1
  Ground_71/1 -> CarCfgForClimaCtrl_CarCfgForRoofHatch_write/1
  Ground_72/1 -> CarCfgForClimaCtrl_CarCfgForSeatHeatgCal_write/1
  Ground_73/1 -> CarCfgForClimaCtrl_CarCfgForSeatHeatgForRowFirst_write/1
  Ground_74/1 -> CarCfgForClimaCtrl_CarCfgForSeatHeatgForRowSec_write/1
  Ground_75/1 -> CarCfgForClimaCtrl_CarCfgForSeatVentnForRowFirst_write/1
  Ground_76/1 -> CarCfgForClimaCtrl_CarCfgForSeatVentnForRowSec_write/1
  Ground_77/1 -> CarCfgForClimaCtrl_CarCfgForSecRowVentnCenter_write/1
  Ground_78/1 -> CarCfgForClimaCtrl_CarCfgForSecRowVentnSide_write/1
  Ground_79/1 -> CarCfgForClimaCtrl_CarCfgForSmallFlowCyc_write/1
  Ground_80/1 -> CarCfgForClimaCtrl_CarCfgForSmkSnsr_write/1
  Ground_81/1 -> CarCfgForClimaCtrl_CarCfgForSnsrForCmptmtT_write/1
  Ground_82/1 -> CarCfgForClimaCtrl_CarCfgForSnsrHumPrsnt_write/1
  Ground_83/1 -> CarCfgForClimaCtrl_CarCfgForStartDevice_write/1
  Ground_84/1 -> CarCfgForClimaCtrl_CarCfgForSteerWhlHeatg_write/1
  Ground_85/1 -> CarCfgForClimaCtrl_CarCfgForSteerWhlPosn_write/1
  Ground_86/1 -> CarCfgForClimaCtrl_CarCfgForSunSnsr_write/1
  Ground_87/1 -> CarCfgForClimaCtrl_CarCfgForTelmNodTyp_write/1
  Ground_88/1 -> CarCfgForClimaCtrl_CarCfgForThermMngt_write/1
  Ground_89/1 -> CarCfgForClimaCtrl_CarCfgForVehTyp_write/1
  Ground_90/1 -> CarCfgForClimaCtrl_CarCfgForVgaMotorExistSts_write/1
  Ground_91/1 -> CarCfgForClimaCtrl_CarCfgForWindHeatd_write/1
  Ground_92/1 -> HvacCfgForClimaCtrl_HvacCfgForHvacFrontBlowerType_write/1
  Ground_93/1 -> HvacCfgForClimaCtrl_HvacCfgForHvacMiscExistSts_write/1
  Ground_94/1 -> HvacCfgForClimaCtrl_HvacCfgForHvacMotorExistSts_write/1
  Ground_95/1 -> HvacCfgForClimaCtrl_HvacCfgForHvacSensorExistSts_write/1
  Ground_96/1 -> SysServCarCfgSts_SysServCarID_write/1
  Ground_97/1 -> SysServCarCfgSts_SysServCCPAQSSts_write/1
  Ground_98/1 -> SysServCarCfgSts_SysServCCPBGMNum_write/1
  Ground_99/1 -> SysServCarCfgSts_SysServCCPHEXSensorSts_write/1
  Ground_100/1 -> SysServCarCfgSts_SysServCCPLin2AFUSts_write/1
  Ground_101/1 -> SysServCarCfgSts_SysServCCPLin2AIUSts_write/1
  Ground_102/1 -> SysServCarCfgSts_SysServCCPLin2CDSSts_write/1
  Ground_103/1 -> SysServCarCfgSts_SysServCCPLin2PMSISts_write/1
  Ground_104/1 -> SysServCarCfgSts_SysServCCPLin3VGASts_write/1
  Ground_105/1 -> SysServCarCfgSts_SysServDUAirVentSnrSts_write/1
  Ground_106/1 -> SysServCarCfgSts_SysServDUBlowerPwmSignSts_write/1
  Ground_107/1 -> SysServCarCfgSts_SysServDUBlowerPwmSts_write/1
  Ground_108/1 -> SysServCarCfgSts_SysServDUDCDefrostSts_write/1
  Ground_109/1 -> SysServCarCfgSts_SysServDUDCFLMdeSts_write/1
  Ground_110/1 -> SysServCarCfgSts_SysServDUDCModeSts_write/1
  Ground_111/1 -> SysServCarCfgSts_SysServDUDCRecSts_write/1
  Ground_112/1 -> SysServCarCfgSts_SysServDUDCSModeSts_write/1
  Ground_113/1 -> SysServCarCfgSts_SysServDUDCSTLSts_write/1
  Ground_114/1 -> SysServCarCfgSts_SysServDUDCTLSts_write/1
  Ground_115/1 -> SysServCarCfgSts_SysServDUDCTRSts_write/1
  Ground_116/1 -> SysServCarCfgSts_SysServDUFLFootSts_write/1
  Ground_117/1 -> SysServCarCfgSts_SysServDUFLVentSts_write/1
  Ground_118/1 -> SysServCarCfgSts_SysServDUFRFootSts_write/1
  Ground_119/1 -> SysServCarCfgSts_SysServDUFRVentSts_write/1
  Ground_120/1 -> SysServCarCfgSts_SysServDUIncarMotorSts_write/1
  Ground_121/1 -> SysServCarCfgSts_SysServDULin1HBMFSts_write/1
  Ground_122/1 -> SysServCarCfgSts_SysServDULin4HFAFSts_write/1
  Ground_123/1 -> SysServCarCfgSts_SysServDUSFLFootSts_write/1
  Ground_124/1 -> SysServCarCfgSts_SysServDUSFLVentSts_write/1
  Ground_125/1 -> SysServCarCfgSts_SysServDUSFRFootSts_write/1
  Ground_126/1 -> SysServCarCfgSts_SysServDUSFRVentSts_write/1
  Ground_127/1 -> SysServCarCfgSts_SysServDUSnsrForFCmptmtTSts_write/1
  Ground_128/1 -> SysServCarCfgSts_SysServDUSnsrForRair_write/1
  Ground_129/1 -> SysServCarCfgSts_SysServDUSnsrForRCmptmtTSts_write/1
  Ground_130/1 -> SysServEcuSlpDelayFinish_EcuSlpDelayFinishFlg_write/1
  Ground_131/1 -> dp_LifeCycle_LifeCycle_write/1
